simulink model "Final_V2"  (R2024a) (continued)
== system Final_V2/The whole system/Battery Pack == (continued)
  wires:
  Cell_14/2(Temp_Cell) -> [Temp_slave_1_cell_14]
  Cell_14/1(Real_SOC_Cell) -> [Real_SOC_slave_1_cell_14]
  Cell_13/1(Real_SOC_Cell) -> [Real_SOC_slave_1_cell_13]
  Cell_13/2(Temp_Cell) -> [Temp_slave_1_cell_13]
  Cell_11/1(Real_SOC_Cell) -> [Real_SOC_slave_1_cell_11]
  Cell_11/2(Temp_Cell) -> [Temp_slave_1_cell_11]
  Cell_12/1(Real_SOC_Cell) -> [Real_SOC_slave_1_cell_12]
  Cell_12/2(Temp_Cell) -> [Temp_slave_1_cell_12]
  Cell_8/2(Temp_Cell) -> [Temp_slave_1_cell_8]
  Cell_8/1(Real_SOC_Cell) -> [Real_SOC_slave_1_cell_8]
  Cell_7/2(Temp_Cell) -> [Temp_slave_1_cell_7]
  Cell_7/1(Real_SOC_Cell) -> [Real_SOC_slave_1_cell_7]
  Cell_9/2(Temp_Cell) -> [Temp_slave_1_cell_9]
  Cell_9/1(Real_SOC_Cell) -> [Real_SOC_slave_1_cell_9]
  Cell_10/1(Real_SOC_Cell) -> [Real_SOC_slave_1_cell_10]
  Cell_10/2(Temp_Cell) -> [Temp_slave_1_cell_10]
  Cell_2/2(Temp_Cell) -> [Temp_slave_1_cell_2]
  Cell_2/1(Real_SOC_Cell) -> [Real_SOC_slave_1_cell_2]
  Cell_1/1(Real_SOC_Cell) -> [Real_SOC_slave_1_cell_1]
  Cell_1/2(Temp_Cell) -> [Temp_slave_1_cell_1]
  Cell_4/2(Temp_Cell) -> [Temp_slave_1_cell_4]
  Cell_4/1(Real_SOC_Cell) -> [Real_SOC_slave_1_cell_4]
  Cell_3/2(Temp_Cell) -> [Temp_slave_1_cell_3]
  Cell_3/1(Real_SOC_Cell) -> [Real_SOC_slave_1_cell_3]
  Cell_5/2(Temp_Cell) -> [Temp_slave_1_cell_5]
  Cell_5/1(Real_SOC_Cell) -> [Real_SOC_slave_1_cell_5]
  Cell_6/1(Real_SOC_Cell) -> [Real_SOC_slave_1_cell_6]
  Cell_6/2(Temp_Cell) -> [Temp_slave_1_cell_6]
  Constant1/1 -> Add/1
  ? -> Add/2
  Add/1 -> Simulink-PS Converter/1
  Bus Creator/1 -> [Real_SOCs_Vector]  "Real_SOCs_Vector"
  Average5/1(Temp.1&2) -> Temp._Sensor/7(in_Temp.1&2)
  Average7/1(Temp.3&4) -> Temp._Sensor/6(in_Temp.3&4)
  Average4/1(Temp.5&6) -> Temp._Sensor/5(in_Temp.5&6)
  Average3/1(Temp.9&10) -> Temp._Sensor/3(in_Temp.9&10)
  Average2/1(Temp.7&8) -> Temp._Sensor/4(in_Temp.7&8)
  Average1/1(Temp.11&12) -> Temp._Sensor/2(in_Temp.11&12)
  Temp._Sensor/1(Temp.1&2) -> Temp.1&2/1
  Temp._Sensor/2(Temp.3&4) -> Temp.3&4/1
  Temp._Sensor/3(Temp.5&6) -> Temp.5&6/1
  Temp._Sensor/4(Temp.7&8) -> Temp.7&8/1
  Temp._Sensor/5(Temp.9&10) -> Temp.9&10/1
  Temp._Sensor/6(Temp.11&12) -> Temp.11&12/1
  Temp._Sensor/7(Temp.13&14) -> Temp.13&14/1
  Average6/1(Temp.13&14) -> Temp._Sensor/1(in_Temp.13&14)
  [Temp_slave_1_cell_14] -> Average6/1(In1)  "Temp_slave_1_cell_14"
  [Temp_slave_1_cell_13] -> Average6/2(In2)  "Temp_slave_1_cell_13"
  [Temp_slave_1_cell_12] -> Average1/1(In1)  "Temp_slave_1_cell_12"
  [Temp_slave_1_cell_11] -> Average1/2(In2)  "Temp_slave_1_cell_11"
  [Temp_slave_1_cell_10] -> Average3/1(In1)  "Temp_slave_1_cell_10"
  [Temp_slave_1_cell_9] -> Average3/2(In2)  "Temp_slave_1_cell_9"
  [Temp_slave_1_cell_8] -> Average2/1(In1)  "Temp_slave_1_cell_8"
  [Temp_slave_1_cell_7] -> Average2/2(In2)  "Temp_slave_1_cell_7"
  [Temp_slave_1_cell_6] -> Average4/1(In1)  "Temp_slave_1_cell_6"
  [Temp_slave_1_cell_5] -> Average4/2(In2)  "Temp_slave_1_cell_5"
  [Temp_slave_1_cell_4] -> Average7/1(In1)  "Temp_slave_1_cell_4"
  [Temp_slave_1_cell_3] -> Average7/2(In2)  "Temp_slave_1_cell_3"
  [Temp_slave_1_cell_2] -> Average5/1(In1)  "Temp_slave_1_cell_2"
  [Temp_slave_1_cell_1] -> Average5/2(In2)  "Temp_slave_1_cell_1"
  [Real_SOC_slave_1_cell_1] -> Bus Creator/1  "Real_SOC_slave_1_cell_1"
  [Real_SOC_slave_1_cell_2] -> Bus Creator/2  "Real_SOC_slave_1_cell_2"
  [Real_SOC_slave_1_cell_3] -> Bus Creator/3  "Real_SOC_slave_1_cell_3"
  [Real_SOC_slave_1_cell_4] -> Bus Creator/4  "Real_SOC_slave_1_cell_4"
  [Real_SOC_slave_1_cell_5] -> Bus Creator/5  "Real_SOC_slave_1_cell_5"
  [Real_SOC_slave_1_cell_6] -> Bus Creator/6  "Real_SOC_slave_1_cell_6"
  [Real_SOC_slave_1_cell_7] -> Bus Creator/7  "Real_SOC_slave_1_cell_7"
  [Real_SOC_slave_1_cell_8] -> Bus Creator/8  "Real_SOC_slave_1_cell_8"
  [Real_SOC_slave_1_cell_9] -> Bus Creator/9  "Real_SOC_slave_1_cell_9"
  [Real_SOC_slave_1_cell_10] -> Bus Creator/10  "Real_SOC_slave_1_cell_10"
  [Real_SOC_slave_1_cell_11] -> Bus Creator/11  "Real_SOC_slave_1_cell_11"
  [Real_SOC_slave_1_cell_12] -> Bus Creator/12  "Real_SOC_slave_1_cell_12"
  [Real_SOC_slave_1_cell_13] -> Bus Creator/13  "Real_SOC_slave_1_cell_13"
  [Real_SOC_slave_1_cell_14] -> Bus Creator/14  "Real_SOC_slave_1_cell_14"
  connections:
  Cell_1/LConn2 -- Cell_2/LConn1
  Cell_2/LConn2 -- Cell_3/LConn1
  Cell_3/LConn2 -- Cell_4/LConn1
  Cell_1/RConn2 -- CT_Cell_2/RConn1 -- Cell_2/RConn1
  Cell_2/RConn2 -- CT_Cell_3/RConn1 -- Cell_3/RConn1
  Cell_3/RConn2 -- CT_Cell_4/RConn1 -- Cell_4/RConn1
  Cell_4/RConn2 -- CT_Cell_5/RConn1 -- Cell_5/RConn1
  Cell_4/LConn2 -- Cell_5/LConn1
  Cell_5/RConn2 -- CT_Cell_6/RConn1 -- Cell_6/RConn1
  Cell_5/LConn2 -- Cell_6/LConn1
  Cell_6/RConn2 -- CT_Cell_7/RConn1 -- Cell_7/RConn1
  Cell_6/LConn2 -- Cell_7/LConn1
  Cell_7/LConn2 -- Cell_8/LConn1
  Cell_7/RConn2 -- CT_Cell_8/RConn1 -- Cell_8/RConn1
  Cell_8/RConn2 -- CT_Cell_9/RConn1 -- Cell_9/RConn1
  Cell_8/LConn2 -- Cell_9/LConn1
  Cell_9/LConn2 -- Cell_10/LConn1
  Cell_9/RConn2 -- CT_Cell_10/RConn1 -- Cell_10/RConn1
  Cell_10/RConn2 -- CT_Cell_11/RConn1 -- Cell_11/RConn1
  Cell_10/LConn2 -- Cell_11/LConn1
  Cell_11/LConn2 -- Cell_12/LConn1
  Cell_11/RConn2 -- CT_Cell_12/RConn1 -- Cell_12/RConn1
  Cell_12/RConn2 -- CT_Cell_13/RConn1 -- Cell_13/RConn1
  Cell_13/RConn2 -- CT_Cell_14/RConn1 -- Cell_14/RConn1
  Cell_12/LConn2 -- Cell_13/LConn1
  Cell_13/LConn2 -- Cell_14/LConn1
  Perfect Insulator1/LConn1 -- Cell_14/LConn2
  CT_Cell_1/RConn1 -- Pack +/RConn1 -- Cell_1/RConn1
  CT_Cell_-14/RConn1 -- Pack -/RConn1 -- Electrical Reference/LConn1 -- Solver Configuration1/RConn1 -- Cell_14/RConn2
  Thermal Reference/LConn1 -- Controlled Temperature Source/RConn2
  Simulink-PS Converter/RConn1 -- Controlled Temperature Source/RConn1
  Controlled Temperature Source/LConn1 -- Cell_1/LConn1

== system Final_V2/The whole system/Charger ==
  Bus Selector : BusSelector  OutputSignals=User_State.Charging_Current_Rqst
  Cast To Double : DataTypeConversion  OutDataTypeStr=double  SaturateOnIntegerOverflow=off  RndMeth=Floor
  Constant : Constant  Value=0
  Controlled Current Source : fl_lib/Electrical/Electrical Sources/Controlled Current Source
  Simulink-PS Converter : nesl_utility/Simulink-PS Converter  PhysicalDomain=network_engine_domain  SubClassName=ps_input  LeftPortType=input  RightPortType=output  PseudoPeriodic=off  Frequencies=[]  NoiseDistribution=none  NoiseParameters=[]  Unit=1  AffineConversion=off  FilteringAndDerivatives=provide  SimscapeFilterOrder=1  InputFilterTimeConstant=0.001  UdotUserProvided=0
  Switch : Switch  Criteria=u2 > Threshold  SaturateOnIntegerOverflow=off  InputSameDT=off
  Charger - : PMIOPort  Side=Left
  Charger + : PMIOPort  Port=2  Side=Left
  wires:
  [User_Data] -> Bus Selector/1
  Cast To Double/1 -> Simulink-PS Converter/1
  Switch/1 -> Cast To Double/1
  [Enable_Charger] -> Switch/2
  Constant/1 -> Switch/3
  Bus Selector/1 -> Switch/1  "<Charging_Current_Rqst>"
  connections:
  Charger -/RConn1 -- Controlled Current Source/RConn2
  Charger +/RConn1 -- Controlled Current Source/LConn1
  Simulink-PS Converter/RConn1 -- Controlled Current Source/RConn1

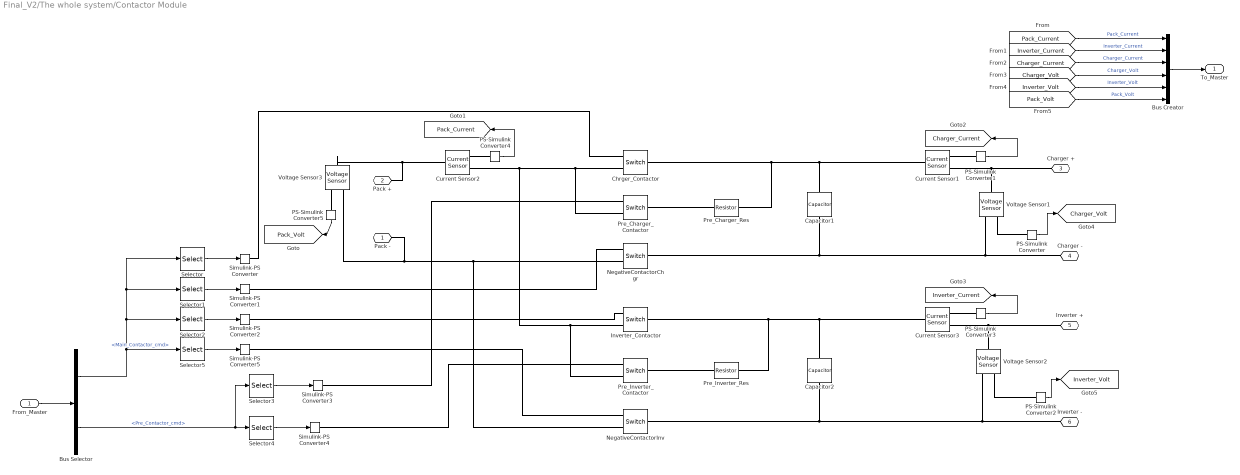
== system Final_V2/The whole system/Contactor Module ==
  From_Master : Inport
  Bus Creator : BusCreator  Inputs=6
  Bus Selector : BusSelector  OutputSignals=Main_Contactor_cmd,Pre_Contactor_cmd
  Capacitor1 : ee_lib/Passive/Capacitor  cap_option=ee.enum.passive.capacitor.capOption.const  c=20 mF  Cvec=[1e-05, 1e-06] F  Vvec=[0, 10] V  CV_table_symmetry=1  f_vec=[1, 10] kHz  DFext_vec=[.8, 1.2]  enable_C_tol=0  averaging_period=0 s  C_tol=5  tol_distribution=1  nSigma=4  r=1e-9 Ohm  g=0 1/Ohm  maxPermissibleTemperature=85 degC  tFailOnTemperature=1 s  enable_operating_limits=0  operating_limit_action=2  max_working_voltage_range=[-25, 25] V  power_rating=1 W  maxTemperature=85 degC  thermalEnable=false  thermalMass=1 J/K  enable_faults=ee.enum.enable.no  fault_action=simscape.enum.assert.action.none  Cscale_faulted=100  R_faulted=1e-3 Ohm  G_faulted=0 1/Ohm  tau_fault=1e-3 s  fault_trigger=ee.enum.faults.faultTrigger.temporal  t_fault=1 s  max_permissible_voltage_range=[-100, 100] V  t_fail_on_voltage=1 s  i_specify=off  i_priority=None  i=0 A  i_nominal_specify=off  i_nominal_value=1  i_nominal_unit=A  v_specify=off  v_priority=None  v=0 V  v_nominal_specify=off  v_nominal_value=1  v_nominal_unit=V  vc_specify=off  vc_priority=High  vc=0 V  vc_nominal_specify=off  vc_nominal_value=1  vc_nominal_unit=V  T_specify=off  T_priority=None  T=298.15 K  T_nominal_specify=off  T_nominal_value=1  T_nominal_unit=K
  Capacitor2 : ee_lib/Passive/Capacitor  cap_option=ee.enum.passive.capacitor.capOption.const  c=20 mF  Cvec=[1e-05, 1e-06] F  Vvec=[0, 10] V  CV_table_symmetry=1  f_vec=[1, 10] kHz  DFext_vec=[.8, 1.2]  enable_C_tol=0  averaging_period=0 s  C_tol=5  tol_distribution=1  nSigma=4  r=1e-9 Ohm  g=0 1/Ohm  maxPermissibleTemperature=85 degC  tFailOnTemperature=1 s  enable_operating_limits=0  operating_limit_action=2  max_working_voltage_range=[-25, 25] V  power_rating=1 W  maxTemperature=85 degC  thermalEnable=false  thermalMass=1 J/K  enable_faults=ee.enum.enable.no  fault_action=simscape.enum.assert.action.none  Cscale_faulted=100  R_faulted=1e-3 Ohm  G_faulted=0 1/Ohm  tau_fault=1e-3 s  fault_trigger=ee.enum.faults.faultTrigger.temporal  t_fault=1 s  max_permissible_voltage_range=[-100, 100] V  t_fail_on_voltage=1 s  i_specify=off  i_priority=None  i=0 A  i_nominal_specify=off  i_nominal_value=1  i_nominal_unit=A  v_specify=off  v_priority=None  v=0 V  v_nominal_specify=off  v_nominal_value=1  v_nominal_unit=V  vc_specify=off  vc_priority=High  vc=0 V  vc_nominal_specify=off  vc_nominal_value=1  vc_nominal_unit=V  T_specify=off  T_priority=None  T=298.15 K  T_nominal_specify=off  T_nominal_value=1  T_nominal_unit=K
  Chrger_Contactor : fl_lib/Electrical/Electrical Elements/Switch  R_closed=0.01 Ohm  G_open=1e-8 1/Ohm  Threshold=0.5  i_specify=off  i_priority=None  i=0 A  i_nominal_specify=off  i_nominal_value=1  i_nominal_unit=A  v_specify=off  v_priority=None  v=0 V  v_nominal_specify=off  v_nominal_value=1  v_nominal_unit=V
  Current Sensor1 : fl_lib/Electrical/Electrical Sensors/Current Sensor
  Current Sensor2 : fl_lib/Electrical/Electrical Sensors/Current Sensor
  Current Sensor3 : fl_lib/Electrical/Electrical Sensors/Current Sensor
  Inverter_Contactor : fl_lib/Electrical/Electrical Elements/Switch  R_closed=0.01 Ohm  G_open=1e-8 1/Ohm  Threshold=0.5  i_specify=off  i_priority=None  i=0 A  i_nominal_specify=off  i_nominal_value=1  i_nominal_unit=A  v_specify=off  v_priority=None  v=0 V  v_nominal_specify=off  v_nominal_value=1  v_nominal_unit=V
  NegativeContactorChgr : fl_lib/Electrical/Electrical Elements/Switch  R_closed=0.001 Ohm  G_open=1e-6 1/Ohm  Threshold=0.5  i_specify=off  i_priority=None  i=0 A  i_nominal_specify=off  i_nominal_value=1  i_nominal_unit=A  v_specify=off  v_priority=None  v=0 V  v_nominal_specify=off  v_nominal_value=1  v_nominal_unit=V
  NegativeContactorInv : fl_lib/Electrical/Electrical Elements/Switch  R_closed=0.01 Ohm  G_open=1e-8 1/Ohm  Threshold=0.5  i_specify=off  i_priority=None  i=0 A  i_nominal_specify=off  i_nominal_value=1  i_nominal_unit=A  v_specify=off  v_priority=None  v=0 V  v_nominal_specify=off  v_nominal_value=1  v_nominal_unit=V
  PS-Simulink Converter{,1..5} (6 alike) : nesl_utility/PS-Simulink Converter  PhysicalDomain=network_engine_domain  SubClassName=ps_output  LeftPortType=input  RightPortType=output  PseudoPeriodic=off  Frequencies=[]  VectorFormat=1-D array  Unit=inherit  AffineConversion=off
  Pre_Charger_Contactor : fl_lib/Electrical/Electrical Elements/Switch  R_closed=0.01 Ohm  G_open=1e-8 1/Ohm  Threshold=0.5  i_specify=off  i_priority=None  i=0 A  i_nominal_specify=off  i_nominal_value=1  i_nominal_unit=A  v_specify=off  v_priority=None  v=0 V  v_nominal_specify=off  v_nominal_value=1  v_nominal_unit=V
  Pre_Charger_Res : fl_lib/Electrical/Electrical Elements/Resistor  R=1 Ohm  i_specify=off  i_priority=None  i=0 A  i_nominal_specify=off  i_nominal_value=1  i_nominal_unit=A  v_specify=off  v_priority=None  v=0 V  v_nominal_specify=off  v_nominal_value=1  v_nominal_unit=V
  Pre_Inverter_Contactor : fl_lib/Electrical/Electrical Elements/Switch  R_closed=0.01 Ohm  G_open=1e-8 1/Ohm  Threshold=0.5  i_specify=off  i_priority=None  i=0 A  i_nominal_specify=off  i_nominal_value=1  i_nominal_unit=A  v_specify=off  v_priority=None  v=0 V  v_nominal_specify=off  v_nominal_value=1  v_nominal_unit=V
  Pre_Inverter_Res : fl_lib/Electrical/Electrical Elements/Resistor  R=1 Ohm  i_specify=off  i_priority=None  i=0 A  i_nominal_specify=off  i_nominal_value=1  i_nominal_unit=A  v_specify=off  v_priority=None  v=0 V  v_nominal_specify=off  v_nominal_value=1  v_nominal_unit=V
  Selector : Selector  InputPortWidth=4  IndexOptions=Index vector (dialog)  Indices=1  OutputSizes=1
  Selector1 : Selector  InputPortWidth=4  IndexOptions=Index vector (dialog)  Indices=2  OutputSizes=1
  Selector2 : Selector  InputPortWidth=4  IndexOptions=Index vector (dialog)  Indices=3  OutputSizes=1
  Selector3 : Selector  InputPortWidth=2  IndexOptions=Index vector (dialog)  Indices=1  OutputSizes=1
  Selector4 : Selector  InputPortWidth=2  IndexOptions=Index vector (dialog)  Indices=2  OutputSizes=1
  Selector5 : Selector  InputPortWidth=4  IndexOptions=Index vector (dialog)  Indices=4  OutputSizes=1
  Simulink-PS Converter{,1..5} (6 alike) : nesl_utility/Simulink-PS Converter  PhysicalDomain=network_engine_domain  SubClassName=ps_input  LeftPortType=input  RightPortType=output  PseudoPeriodic=off  Frequencies=[]  NoiseDistribution=none  NoiseParameters=[]  Unit=1  AffineConversion=off  FilteringAndDerivatives=provide  SimscapeFilterOrder=1  InputFilterTimeConstant=0.001  UdotUserProvided=0
  Voltage Sensor1 : fl_lib/Electrical/Electrical Sensors/Voltage Sensor
  Voltage Sensor2 : fl_lib/Electrical/Electrical Sensors/Voltage Sensor
  Voltage Sensor3 : fl_lib/Electrical/Electrical Sensors/Voltage Sensor
  Pack - : PMIOPort  Side=Left
  Pack + : PMIOPort  Port=2  Side=Left
  Charger + : PMIOPort  Port=3  Side=Right
  Charger - : PMIOPort  Port=4  Side=Right
  Inverter + : PMIOPort  Port=5  Side=Right
  Inverter - : PMIOPort  Port=6  Side=Right
  To_Master : Outport
  tag scope: Charger_Current=global, Charger_Volt=global, Inverter_Current=global, Inverter_Volt=global, Pack_Current=global, Pack_Volt=global
  wires:
  PS-Simulink Converter4/1 -> [Pack_Current]
  PS-Simulink Converter1/1 -> [Charger_Current]
  PS-Simulink Converter5/1 -> [Pack_Volt]
  PS-Simulink Converter3/1 -> [Inverter_Current]
  Selector/1 -> Simulink-PS Converter/1
  Selector2/1 -> Simulink-PS Converter2/1
  Selector1/1 -> Simulink-PS Converter1/1
  Selector3/1 -> Simulink-PS Converter3/1
  Selector4/1 -> Simulink-PS Converter4/1
  Bus Selector/2 -> Selector4/1, Selector3/1  "<Pre_Contactor_cmd>"
  From_Master/1 -> Bus Selector/1
  Bus Creator/1 -> To_Master/1
  PS-Simulink Converter/1 -> [Charger_Volt]
  PS-Simulink Converter2/1 -> [Inverter_Volt]
  [Pack_Volt] -> Bus Creator/6  "Pack_Volt"
  [Inverter_Volt] -> Bus Creator/5  "Inverter_Volt"
  [Charger_Volt] -> Bus Creator/4  "Charger_Volt"
  [Charger_Current] -> Bus Creator/3  "Charger_Current"
  [Inverter_Current] -> Bus Creator/2  "Inverter_Current"
  [Pack_Current] -> Bus Creator/1  "Pack_Current"
  Bus Selector/1 -> Selector5/1, Selector/1, Selector1/1, Selector2/1  "<Main_Contactor_cmd>"
  Selector5/1 -> Simulink-PS Converter5/1
  connections:
  Current Sensor2/RConn1 -- PS-Simulink Converter4/LConn1
  Current Sensor1/RConn1 -- PS-Simulink Converter1/LConn1
  PS-Simulink Converter3/LConn1 -- Current Sensor3/RConn1
  Voltage Sensor3/LConn1 -- Current Sensor2/LConn1 -- Pack +/RConn1
  Pack -/RConn1 -- NegativeContactorInv/RConn2 -- NegativeContactorChgr/RConn2 -- Voltage Sensor3/RConn2
  Charger -/RConn1 -- Capacitor1/RConn1 -- NegativeContactorChgr/LConn1 -- Voltage Sensor1/RConn2
  Voltage Sensor2/RConn2 -- Capacitor2/RConn1 -- NegativeContactorInv/LConn1 -- Inverter -/RConn1
  Current Sensor3/RConn2 -- Voltage Sensor2/LConn1 -- Inverter +/RConn1
  Current Sensor1/RConn2 -- Voltage Sensor1/LConn1 -- Charger +/RConn1
  Chrger_Contactor/RConn1 -- Simulink-PS Converter/RConn1
  Pre_Charger_Contactor/RConn1 -- Simulink-PS Converter3/RConn1
  Pre_Inverter_Contactor/RConn1 -- Simulink-PS Converter4/RConn1
  NegativeContactorChgr/RConn1 -- Simulink-PS Converter1/RConn1
  Inverter_Contactor/RConn2 -- Pre_Inverter_Contactor/RConn2 -- Current Sensor2/RConn2 -- Pre_Charger_Contactor/RConn2 -- Chrger_Contactor/RConn2
  Inverter_Contactor/RConn1 -- Simulink-PS Converter2/RConn1
  Simulink-PS Converter5/RConn1 -- NegativeContactorInv/RConn1
  PS-Simulink Converter5/LConn1 -- Voltage Sensor3/RConn1
  Pre_Inverter_Contactor/LConn1 -- Pre_Inverter_Res/LConn1
  Pre_Charger_Contactor/LConn1 -- Pre_Charger_Res/LConn1
  Voltage Sensor1/RConn1 -- PS-Simulink Converter/LConn1
  Voltage Sensor2/RConn1 -- PS-Simulink Converter2/LConn1
  Current Sensor1/LConn1 -- Capacitor1/LConn1 -- Chrger_Contactor/LConn1 -- Pre_Charger_Res/RConn1
  Current Sensor3/LConn1 -- Capacitor2/LConn1 -- Inverter_Contactor/LConn1 -- Pre_Inverter_Res/RConn1

== system Final_V2/The whole system/Isolated Network Transceiver ==
  From_Slave : Inport
  From_Master : Inport  Port=2
  To_Master : Outport
  To_Slave : Outport  Port=2
  wires:
  From_Master/1 -> To_Slave/1
  From_Slave/1 -> To_Master/1

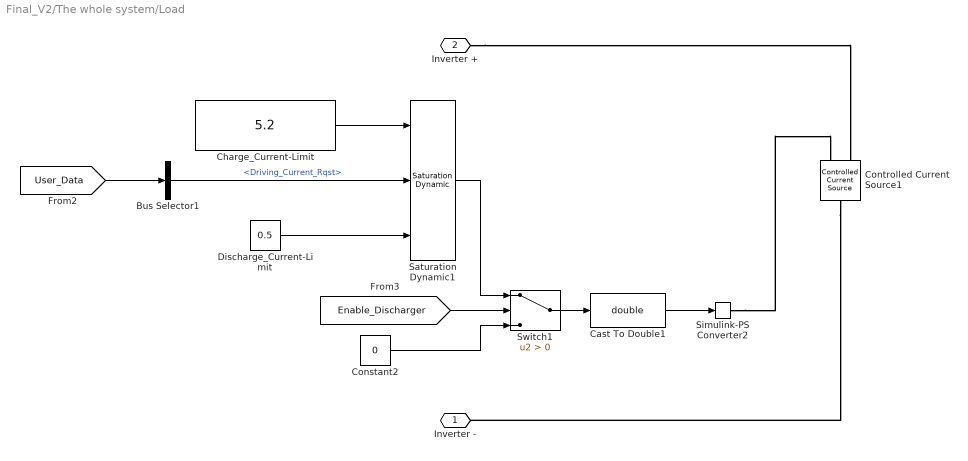
== system Final_V2/The whole system/Load ==
  Bus Selector1 : BusSelector  OutputSignals=User_State.Driving_Current_Rqst
  Cast To Double1 : DataTypeConversion  OutDataTypeStr=double  SaturateOnIntegerOverflow=off  RndMeth=Floor
  Charge_Current-Limit : Constant  Value=5.2
  Constant2 : Constant  Value=0
  Controlled Current Source1 : fl_lib/Electrical/Electrical Sources/Controlled Current Source
  Discharge_Current-Limit : Constant  Value=0.5
  Saturation Dynamic1 : simulink/Discontinuities/Saturation Dynamic  OutMin=[]  OutMax=[]  OutDataTypeStr=Inherit: Same as second input  OutDataType=fixdt(1, 16)  OutScaling=2^-10  DoSatur=off
  Simulink-PS Converter2 : nesl_utility/Simulink-PS Converter  PhysicalDomain=network_engine_domain  SubClassName=ps_input  LeftPortType=input  RightPortType=output  PseudoPeriodic=off  Frequencies=[]  NoiseDistribution=none  NoiseParameters=[]  Unit=1  AffineConversion=off  FilteringAndDerivatives=provide  SimscapeFilterOrder=1  InputFilterTimeConstant=0.001  UdotUserProvided=0
  Switch1 : Switch  Criteria=u2 > Threshold  SaturateOnIntegerOverflow=off  InputSameDT=off
  Inverter - : PMIOPort  Side=Left
  Inverter + : PMIOPort  Port=2  Side=Left
  wires:
  [User_Data] -> Bus Selector1/1
  Discharge_Current-Limit/1 -> Saturation Dynamic1/3
  Saturation Dynamic1/1 -> Switch1/1
  Constant2/1 -> Switch1/3
  Switch1/1 -> Cast To Double1/1
  [Enable_Discharger] -> Switch1/2
  Charge_Current-Limit/1 -> Saturation Dynamic1/1
  Cast To Double1/1 -> Simulink-PS Converter2/1
  Bus Selector1/1 -> Saturation Dynamic1/2  "<Driving_Current_Rqst>"
  connections:
  Controlled Current Source1/LConn1 -- Inverter -/RConn1
  Inverter +/RConn1 -- Controlled Current Source1/RConn2
  Simulink-PS Converter2/RConn1 -- Controlled Current Source1/RConn1

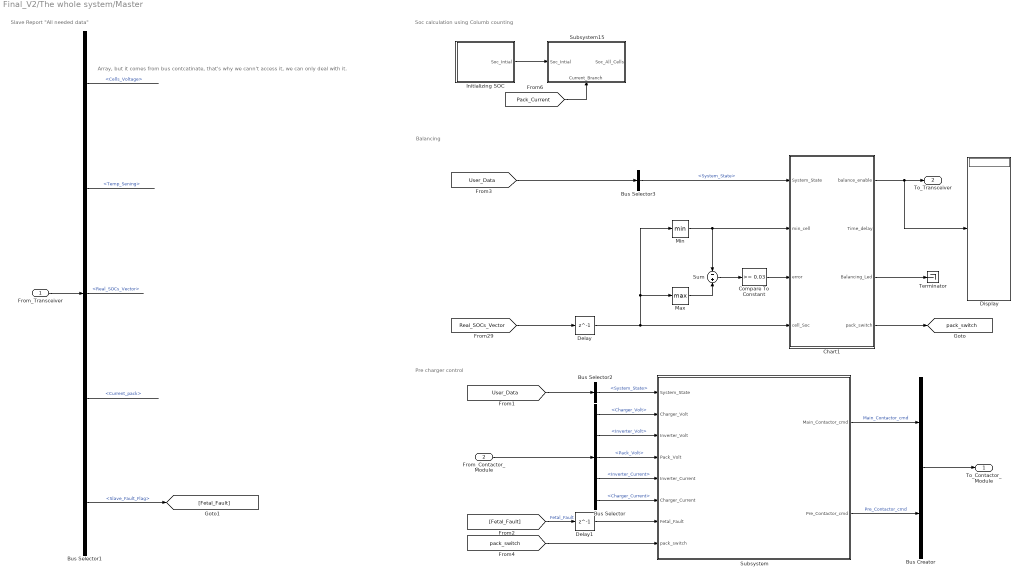
== system Final_V2/The whole system/Master ==
  note: Array, but it comes from bus contcatinate, that's why we cann't access it, we can only deal with it.
  note: Soc calculation using Columb counting
  note: Pre charger control
  note: Balancing
  note: Slave Report "All needed data"
  From_Transceiver : Inport
  From_Contactor_Module : Inport  Port=2
  Bus Creator : BusCreator  Inputs=2
  Bus Selector : BusSelector  OutputSignals=Charger_Volt,Inverter_Volt,Pack_Volt,Inverter_Current,Charger_Current
  Bus Selector1 : BusSelector  OutputSignals=Cells_Voltage,Temp_Sening,Real_SOCs_Vector,Current_pack,Slave_Fault_Flag
  Bus Selector2 : BusSelector  OutputSignals=User_State.System_State
  Bus Selector3 : BusSelector  OutputSignals=User_State.System_State
  Chart1 : Stateflow chart  TreatAsAtomicUnit=on
      settings: PortSchema="{\"entries\":[{\"content\":{\"sides\":[{\"content\":{\"connectorIds\":[\"In2\",\"In4\",\"In3\",\"In1\"]},\"type\":\"ConnectorPlacement.EquallySpacedRectSide\",\"uuid\":\"6f312cc7-79af-4024-8579-3732173f9295\"},{\"content\":{\"connectorIds\":[\"Out1\",\"Out3\",\"Out4\",\"Out2\"],\"side\":\"RIGHT\"},\"type\":\"ConnectorPlacement.EquallySpacedRectSide\",\"uuid\":\"3dc9c74e-fd1a-461c-96fc-bb153941e7b5\"},{\"content\":{\"side\":\"TOP\"},\"type\":\"ConnectorPlacement.EquallySpacedRectSide\",\"uuid\":\"e4504d25-18f4-45f4-b711-d682a6c51dcf\"}]},\"type\":\"ConnectorPlacement.EquallySpacedConnector\",\"uuid\":\"9caf59e1-89f7-43be-b634-7a97cd583662\"}],\"packageUris\":[\"http://schema.mathworks.com/mf0/Connector/R2020b\"],\"version\":\"1.0\"}"
  Compare To Constant : simulink/Logic and Bit Operations/Compare To Constant  relop=>=  const=0.03  OutDataTypeStr=boolean
  Delay : Delay  DelayLength=1
  Delay1 : Delay  DelayLength=1
  Display : Display
  Initializing SOC : Subsystem
  Max : MinMax  Function=max
  Min : MinMax
  Subsystem : Subsystem
  Subsystem15 : Subsystem
      settings: PortSchema="{\"entries\":[{\"content\":{\"sides\":[{\"content\":{\"connectorIds\":[\"In1\"]},\"type\":\"ConnectorPlacement.EquallySpacedRectSide\",\"uuid\":\"04ed98b0-2c8a-4966-9e77-775b47f93202\"},{\"content\":{\"connectorIds\":[\"Out1\"],\"side\":\"RIGHT\"},\"type\":\"ConnectorPlacement.EquallySpacedRectSide\",\"uuid\":\"d1ea0187-eebe-48ef-b874-5671e5114b7f\"},{\"content\":{\"side\":\"TOP\"},\"type\":\"ConnectorPlacement.EquallySpacedRectSide\",\"uuid\":\"d9957a34-6335-44da-afcb-18bb0b6434da\"},{\"content\":{\"connectorIds\":[\"In2\"],\"side\":\"BOTTOM\"},\"type\":\"ConnectorPlacement.EquallySpacedRectSide\",\"uuid\":\"d9902b46-da12-4661-926b-aa768ceeb813\"}]},\"type\":\"ConnectorPlacement.EquallySpacedConnector\",\"uuid\":\"da08e6a5-71fb-48bb-b3e4-5e34c772a1cf\"}],\"packageUris\":[\"http://schema.mathworks.com/mf0/Connector/R2020b\"],\"version\":\"1.0\"}"
  Sum : Sum  Inputs=-+
  Terminator : Terminator
  To_Contactor_Module : Outport
  To_Transceiver : Outport  Port=2
  tag scope: pack_switch=global
  wires:
  [User_Data] -> Bus Selector3/1
  Min/1 -> Chart1/4(min_cell), Sum/1
  Max/1 -> Sum/2
  Sum/1 -> Compare To Constant/1
  Bus Selector3/1 -> Chart1/2(System_State)  "<System_State>"
  [Pack_Current] -> Subsystem15/2(Current_Branch)
  Subsystem/1(Main_Contactor_cmd) -> Bus Creator/1  "Main_Contactor_cmd"
  Bus Selector/1 -> Subsystem/2(Charger_Volt)  "<Charger_Volt>"
  Bus Selector/2 -> Subsystem/3(Inverter_Volt)  "<Inverter_Volt>"
  From_Contactor_Module/1 -> Bus Selector/1
  Bus Selector/3 -> Subsystem/4(Pack_Volt)  "<Pack_Volt>"
  Bus Selector2/1 -> Subsystem/1(System_State)  "<System_State>"
  Subsystem/2(Pre_Contactor_cmd) -> Bus Creator/2  "Pre_Contactor_cmd"
  Bus Creator/1 -> To_Contactor_Module/1
  [User_Data] -> Bus Selector2/1
  Initializing SOC/1(Soc_Intial) -> Subsystem15/1(Soc_Intial)
  Chart1/1(balance_enable) -> Display/1, To_Transceiver/1
  From_Transceiver/1 -> Bus Selector1/1
  Bus Selector1/1 -> ?  "<Cells_Voltage>"
  Bus Selector1/4 -> ?  "<Current_pack>"
  Bus Selector1/5 -> [Fetal_Fault]  "<Slave_Fault_Flag>"
  Compare To Constant/1 -> Chart1/3(error)
  Bus Selector/4 -> Subsystem/5(Inverter_Current)  "<Inverter_Current>"
  Bus Selector/5 -> Subsystem/6(Charger_Current)  "<Charger_Current>"
  Delay/1 -> Min/1, Max/1, Chart1/1(cell_Soc)
  [Fetal_Fault] -> Delay1/1  "Fetal_Fault"
  Delay1/1 -> Subsystem/7(Fetal_Fault)
  Bus Selector1/3 -> ?  "<Real_SOCs_Vector>"
  [Real_SOCs_Vector] -> Delay/1
  Chart1/2(pack_switch) -> [pack_switch]
  Chart1/4(Balancing_Led) -> Terminator/1
  [pack_switch] -> Subsystem/8(pack_switch)
  Bus Selector1/2 -> ?  "<Temp_Sening>"
